simulink model "ofdm_txrx_alamouti"  (Simulink 6.3)
settings: Solver=ode45  StartTime=0.0  StopTime=45000  RelTol=1e-3
InitFcn:
  | ofdm_rx_alamouti_init
StartFcn:
  | ofdm_rx_alamouti_init
values from neighbouring files (symbols this model uses):
  CPLength = 16    [ofdm_tx_alamouti_init.m]
  CRCPolynomial = hex2dec('04c11db7')    [calcTxCRC.m]    (also in ofdm_tx_alamouti_init.m)
  hi = zeros(1,length(cci))    [ofdm_rx_alamouti_init.m]
  hr = zeros(1,length(ccr))    [ofdm_rx_alamouti_init.m]
  max_CP_length = 16    [ofdm_tx_alamouti_init.m]
  max_num_baseRateSymbols = 31    [ofdm_tx_alamouti_init.m]
  max_num_subcarriers = 64    [ofdm_tx_alamouti_init.m]
  max_num_trainingSymbols = 15    [ofdm_tx_alamouti_init.m]
  max_OFDM_symbols = 2047    [ofdm_tx_alamouti_init.m]
  modConstellation_qam16 = 0.75*[1 3 -1 -3]./3    [ofdm_tx_alamouti_init.m]
  modConstellation_qam256 = 0.9375*[3 1 5 7 11 9 13 15 -3 -1 -5 -7 -11 -9 -13 -15]./15    [ofdm_tx_alamouti_init.m]
  modConstellation_qam64 = 0.875*[3 1 5 7 -3 -1 -5 -7]./7    [ofdm_tx_alamouti_init.m]
  modConstellation_qpsk = [1 -1]./sqrt(2)    [ofdm_tx_alamouti_init.m]
  numBaseRateSymbols = 2    [ofdm_tx_alamouti_init.m]
  numFullRateSymbols = numFullRateSymbols + mod(numFullRateSymbols, 2)    [ofdm_tx_alamouti_init.m]
  numSubcarriers = 64    [ofdm_tx_alamouti_init.m]
  numTrainingSymbols = 4    [ofdm_tx_alamouti_init.m]
  pkt_crossCorr_thresh = 0.7    [ofdm_rx_alamouti_init.m]
  pkt_energy_thresh = 0    [ofdm_rx_alamouti_init.m]
  pkt_numPayloadBytes = 24+4+20+20+500    (= 568)    [ofdm_tx_alamouti_init.m]
  pktTiming_count_payloadStart = pktTiming_count_lsStart + pktTiming_ls_num*pktTiming_ls_length    [ofdm_rx_init_packetTimingControl.m]
  preamble = 6*[0 shortsyms_10 longsyms_2]    [ofdm_tx_alamouti_init.m]
  preamble_I = real(preamble)    [ofdm_tx_alamouti_init.m]
  preamble_Q = imag(preamble)    [ofdm_tx_alamouti_init.m]
  RAM_init_size = 4096    [ofdm_tx_alamouti_init.m]
  rx_longCorrCountLoad = 251-16    (= 235)    [ofdm_rx_alamouti_init.m]
  rx_longCorrThresh = 3000    [ofdm_rx_alamouti_init.m]
  RxDataScrambling_Seq = [40 198 78 63 82 173 102 245 48 111 172 115 147 230 216 93 72 65 62 2 205 242 122 90 128 83 105 97 73 10 5 252]    [ofdm_tx_alamouti_init.m]
  simOnly_numSamples = length(preamble)+( (numSubcarriers+CPLength)*(numTrainingSymbols + numBaseRateSymbols + numFullRateSymbols) )    [ofdm_tx_alamouti_init.m]
  train = [train train]    [ofdm_tx_alamouti_init.m]
  TxDataScrambling_Seq = [40 198 78 63 82 173 102 245 48 111 172 115 147 230 216 93 72 65 62 2 205 242 122 90 128 83 105 97 73 10 5 252]    [ofdm_tx_alamouti_init.m]
undefined symbols (not defined in the file or in its folder): CRC_table_gen, gcbh, interpFilter_4, xlBlockLoadCallback
libraries: xbsIndex_r4 x4373, dspmlti4 x4, dspsigops x4, commchan3 x2, simulink x2, commrflib2 x2, commsink2 x2, dspsrcs4 x1
(part 4 of 39)

== system ofdm_txrx_alamouti/OFDM Tx Alamouti/PreCFO ==
  " AI" : Inport
  " AQ" : Inport  Port=2
  " BI" : Inport  Port=3
  " BQ" : Inport  Port=4
  A*B : Subsystem
  A*B1 : Subsystem
  Convert{1..4} (4 alike) : xbsIndex_r4/Convert  arith_type="Signed  (2's comp)"  n_bits=16  bin_pt=15  quantization=Truncate  overflow=Flag as error  latency=0  dbl_ovrd=off  pipeline=off  block_type=convert
      settings: xl_area=[0 0 0 0 0 0 0]  block_version=VER_STRING_GOES_HERE
  Convert5 : xbsIndex_r4/Convert  arith_type=Unsigned  n_bits=32  bin_pt=32  quantization=Truncate  overflow=Wrap  latency=0  dbl_ovrd=off  pipeline=off  block_type=convert
      settings: xl_area=[0 0 0 0 0 0 0]  block_version=VER_STRING_GOES_HERE
  Convert6 : xbsIndex_r4/Convert  arith_type=Boolean  n_bits=32  bin_pt=32  quantization=Truncate  overflow=Wrap  latency=0  dbl_ovrd=off  pipeline=off  block_type=convert
      settings: xl_area=[0 0 0 0 0 0 0]  block_version=VER_STRING_GOES_HERE
  DDS : Subsystem
  Down Sample1 : xbsIndex_r4/Down Sample  sample_ratio=2  sample_phase="Last Value of Frame  (most efficient)"  en=off  latency=1  dbl_ovrd=off  block_type=dsamp
      settings: block_version=VER_STRING_GOES_HERE
  Freq Correction Sinusoids : Scope  NumInputPorts=2
      settings: TimeRange=5000  YMin=-1~-1  YMax=0.4~1  SaveName=ScopeData60
  Gateway Out : xbsIndex_r4/Gateway Out  hdl_port=off  timing_constraint=None  locs_specified=off  LOCs={}  block_type=gatewayout
      settings: infoedit="Gateway out block.  Converts Xilinx fixed point inputs into ouputs of type Simulink integer, double, or fixed point.<P><P>Hardware notes:  In hardware these blocks become top level output ports or are discarded, depending on how they are configured."  xl_area=[0 0 0 0 0 0 0]  block_version=VER_STRING_GOES_HERE
  Gateway Out1 : xbsIndex_r4/Gateway Out  hdl_port=off  timing_constraint=None  locs_specified=off  LOCs={}  block_type=gatewayout
      settings: infoedit="Gateway out block.  Converts Xilinx fixed point inputs into ouputs of type Simulink integer, double, or fixed point.<P><P>Hardware notes:  In hardware these blocks become top level output ports or are discarded, depending on how they are configured."  xl_area=[0 0 0 0 0 0 0]  block_version=VER_STRING_GOES_HERE
  Gateway Out2 : xbsIndex_r4/Gateway Out  hdl_port=off  timing_constraint=None  locs_specified=off  LOCs={}  block_type=gatewayout
      settings: infoedit="Gateway out block.  Converts Xilinx fixed point inputs into ouputs of type Simulink integer, double, or fixed point.<P><P>Hardware notes:  In hardware these blocks become top level output ports or are discarded, depending on how they are configured."  xl_area=[0 0 0 0 0 0 0]  block_version=VER_STRING_GOES_HERE
  Inverter : xbsIndex_r4/Inverter  en=off  latency=0  dbl_ovrd=off  block_type=inv
      settings: block_version=8.2.01
  Logical5 : xbsIndex_r4/Logical  logical_function=OR  inputs=2  en=off  latency=0  precision=Full  arith_type=Unsigned  n_bits=8  bin_pt=2  align_bp=on  dbl_ovrd=off  block_type=logical
      settings: xl_area=[0, 0, 0, 0, 0, 0, 0]  block_version=VER_STRING_GOES_HERE
  Mux2 : Mux  Inputs=2
  AI : Outport
  AQ : Outport  Port=2
  BI : Outport  Port=3
  BQ : Outport  Port=4
  wires:
  A*B1/2(Im) -> Convert4/1
  " BQ"/1 -> A*B1/2(Im[A])
  A*B1/1(Re) -> Convert3/1
  " AQ"/1 -> A*B/2(Im[A])
  A*B/1(Re) -> Convert1/1
  " AI"/1 -> A*B/1(Re[A])
  A*B/2(Im) -> Convert2/1
  Convert1/1 -> AI/1
  Convert2/1 -> AQ/1
  " BI"/1 -> A*B1/1(Re[A])
  Convert3/1 -> BI/1
  Convert4/1 -> BQ/1
  DDS/1(Q) -> A*B/3(Im[B]), A*B1/3(Im[B]), Gateway Out/1
  DDS/2(I) -> Gateway Out1/1, A*B/4(Re[B]), A*B1/4(Re[B])
  [Rx_CFO_PhaseInc] -> Convert5/1
  Convert5/1 -> DDS/1(PhaseInc), Gateway Out2/1
  [TxReset] -> Logical5/1
  Gateway Out1/1 -> Mux2/1
  Gateway Out/1 -> Mux2/2
  Mux2/1 -> Freq Correction Sinusoids/1
  Logical5/1 -> Down Sample1/1
  [TxReg_EnableTxPreCFO] -> Inverter/1
  Convert6/1 -> Logical5/2
  Down Sample1/1 -> DDS/2(Rst)
  Inverter/1 -> Convert6/1
  Gateway Out2/1 -> Freq Correction Sinusoids/2

== system ofdm_txrx_alamouti/OFDM Tx Alamouti/Restarter ==
  StartTx : Inport
  Done : Inport  Port=2
  Convert{,1..5} (6 alike) : xbsIndex_r4/Convert  arith_type=Boolean  n_bits=1  bin_pt=0  quantization=Truncate  overflow=Wrap  latency=0  dbl_ovrd=off  pipeline=off  block_type=convert
      settings: block_version=8.2.02
  Counter : xbsIndex_r4/Counter  cnt_type=Free Running  cnt_to=Inf  operation=Up  start_count=0  cnt_by_val=1  arith_type=Unsigned  n_bits=14  bin_pt=0  load_pin=off  rst=on  en=on  explicit_period=off  period=4  dbl_ovrd=off  use_behavioral_HDL=off  use_rpm=off  block_type=counter
      settings: block_version=8.2.02
  Delay : xbsIndex_r4/Delay  en=off  latency=16  dbl_ovrd=off  reg_retiming=off  block_type=delay
      settings: infoedit=Hardware notes: A delay line is a chain, each link of which is an SRL16 followed by a flip-flop. If register retiming is enabled, the delay line is a chain of flip-flops.  xl_area=[1 1 0 0 0 0 0]  block_version=VER_STRING_GOES_HERE
  Inverter : xbsIndex_r4/Inverter  en=off  latency=0  dbl_ovrd=off  block_type=inv
      settings: block_version=8.2.02
  Inverter1 : xbsIndex_r4/Inverter  en=off  latency=0  dbl_ovrd=off  block_type=inv
      settings: block_version=8.2.02
  Logical1 : xbsIndex_r4/Logical  logical_function=OR  inputs=3  en=off  latency=0  precision=Full  arith_type=Unsigned  n_bits=16  bin_pt=0  align_bp=on  dbl_ovrd=off  block_type=logical
      settings: block_version=VER_STRING_GOES_HERE
  Logical10 : xbsIndex_r4/Logical  logical_function=AND  inputs=2  en=off  latency=0  precision=Full  arith_type=Unsigned  n_bits=16  bin_pt=0  align_bp=on  dbl_ovrd=off  block_type=logical
      settings: block_version=VER_STRING_GOES_HERE
  Logical11 : xbsIndex_r4/Logical  logical_function=AND  inputs=2  en=off  latency=0  precision=Full  arith_type=Unsigned  n_bits=16  bin_pt=0  align_bp=on  dbl_ovrd=off  block_type=logical
      settings: block_version=VER_STRING_GOES_HERE
  Logical2 : xbsIndex_r4/Logical  logical_function=AND  inputs=3  en=off  latency=0  precision=Full  arith_type=Unsigned  n_bits=16  bin_pt=0  align_bp=on  dbl_ovrd=off  block_type=logical
      settings: block_version=VER_STRING_GOES_HERE
  Logical3 : xbsIndex_r4/Logical  logical_function=AND  inputs=2  en=off  latency=0  precision=Full  arith_type=Unsigned  n_bits=16  bin_pt=0  align_bp=on  dbl_ovrd=off  block_type=logical
      settings: block_version=VER_STRING_GOES_HERE
  Logical4 : xbsIndex_r4/Logical  logical_function=OR  inputs=3  en=off  latency=0  precision=Full  arith_type=Unsigned  n_bits=16  bin_pt=0  align_bp=on  dbl_ovrd=off  block_type=logical
      settings: block_version=VER_STRING_GOES_HERE
  Logical5 : xbsIndex_r4/Logical  logical_function=OR  inputs=2  en=off  latency=0  precision=Full  arith_type=Unsigned  n_bits=16  bin_pt=0  align_bp=on  dbl_ovrd=off  block_type=logical
      settings: block_version=VER_STRING_GOES_HERE
  Logical6 : xbsIndex_r4/Logical  logical_function=XOR  inputs=2  en=off  latency=0  precision=Full  arith_type=Unsigned  n_bits=16  bin_pt=0  align_bp=on  dbl_ovrd=off  block_type=logical
      settings: block_version=VER_STRING_GOES_HERE
  Logical7 : xbsIndex_r4/Logical  logical_function=OR  inputs=2  en=off  latency=0  precision=Full  arith_type=Unsigned  n_bits=16  bin_pt=0  align_bp=on  dbl_ovrd=off  block_type=logical
      settings: block_version=VER_STRING_GOES_HERE
  Logical8 : xbsIndex_r4/Logical  logical_function=AND  inputs=3  en=off  latency=0  precision=Full  arith_type=Unsigned  n_bits=16  bin_pt=0  align_bp=on  dbl_ovrd=off  block_type=logical
      settings: block_version=VER_STRING_GOES_HERE
  Posedge1 : Subsystem
  Posedge2 : Subsystem
  Radio_RxEn : xbsIndex_r4/Gateway Out  hdl_port=on  timing_constraint=None  locs_specified=off  LOCs={}  block_type=gatewayout
      settings: infoedit="Gateway out block.  Converts Xilinx fixed point inputs into ouputs of type Simulink integer, double, or fixed point.<P><P>Hardware notes:  In hardware these blocks become top level output ports or are discarded, depending on how they are configured."  xl_area=[0 0 0 0 1 0 0]  block_version=VER_STRING_GOES_HERE
  Radio_TxEn : xbsIndex_r4/Gateway Out  hdl_port=on  timing_constraint=None  locs_specified=off  LOCs={}  block_type=gatewayout
      settings: infoedit="Gateway out block.  Converts Xilinx fixed point inputs into ouputs of type Simulink integer, double, or fixed point.<P><P>Hardware notes:  In hardware these blocks become top level output ports or are discarded, depending on how they are configured."  xl_area=[0 0 0 0 1 0 0]  block_version=VER_STRING_GOES_HERE
  ReTx Control : Scope  NumInputPorts=8
      settings: TimeRange=7000  YMin=0~0~0~0~-1~-1~-1~-1  YMax=40~1~1~1~1~1~1~1  SaveName=ScopeData70
  Relational : xbsIndex_r4/Relational  mode=a=b  en=off  latency=1  dbl_ovrd=off  block_type=relational
      settings: block_version=8.2.02
  SR : Subsystem
  SR1 : Subsystem
  SR2 : Subsystem
  SR3 : Subsystem
  done{1..6} (6 alike) : xbsIndex_r4/Gateway Out  hdl_port=off  timing_constraint=None  locs_specified=off  LOCs={}  block_type=gatewayout
      settings: infoedit="Gateway out block.  Converts Xilinx fixed point inputs into ouputs of type Simulink integer, double, or fixed point.<P><P>Hardware notes:  In hardware these blocks become top level output ports or are discarded, depending on how they are configured."  xl_area=[0 0 0 0 1 0 0]  block_version=VER_STRING_GOES_HERE
  negedge : Subsystem
  Start : Outport
  2ndTx : Outport  Port=2
  tag scope: TxRestarter_DelayPktDone=global
  wires:
  Counter/1 -> Relational/2
  [TxReg_2ndTxDelay] -> Relational/1
  Logical1/1 -> SR/1(R)
  StartTx/1 -> done4/1, Posedge2/1(D)
  [TxReset] -> Logical1/3
  SR/1(Q) -> Counter/2, Inverter/1
  Posedge1/1(Q) -> Logical2/1, Logical5/2, SR2/2(R)
  [TxReg_2ndTxEn] -> Convert1/1
  Inverter/1 -> Counter/1
  Relational/1 -> Logical1/1, Logical3/1, Logical11/1
  [TxReg_2ndTxEn] -> Convert/1
  Logical3/1 -> Logical4/2, SR1/1(S)
  Logical4/1 -> Start/1, done1/1
  Convert1/1 -> Logical2/2, Logical10/1
  Logical5/1 -> SR1/2(R)
  [TxReset] -> Logical5/1
  SR1/1(Q) -> Logical6/1, Inverter1/1
  Logical6/1 -> 2ndTx/1, done2/1
  [TxReg_2ndTxAntB] -> Convert2/1
  Convert2/1 -> Logical6/2
  Inverter1/1 -> Logical2/3, Logical8/1, Logical10/2
  Done/1 -> Posedge1/1(D), done6/1
  done4/1 -> ReTx Control/1  "Ext Start"
  done1/1 -> ReTx Control/2  "Start Out"
  done2/1 -> ReTx Control/3  "2nd Tx Out"
  done3/1 -> ReTx Control/4  "DF Relay ReTx Start"
  done5/1 -> ReTx Control/5  "AF Relay ReTx Start"
  done6/1 -> ReTx Control/6  "Tx Done"
  Radio_RxEn/1 -> ReTx Control/8  "Radio_RxEn"
  [Rx_GoodPktInterrupt] -> Convert4/1
  [TxReg_RelayTxEn] -> Convert3/1
  Logical2/1 -> Logical7/1, done5/1
  Logical8/1 -> Logical7/2, done3/1
  Logical7/1 -> SR/2(S)
  Convert/1 -> Logical3/2
  Convert3/1 -> Logical8/2
  Convert4/1 -> Logical8/3
  [TxReg_RelayTxEn] -> Convert5/1
  Convert5/1 -> Logical11/2
  Posedge2/1(Q) -> Logical4/1, Logical1/2
  Logical10/1 -> [TxRestarter_DelayPktDone]
  SR2/1(Q) -> Radio_TxEn/1, negedge/1(D)
  Logical11/1 -> Logical4/3, SR2/1(S), SR3/2(R)
  negedge/1(Q) -> Delay/1
  Delay/1 -> SR3/1(S)
  SR3/1(Q) -> Radio_RxEn/1
  Radio_TxEn/1 -> ReTx Control/7  "Radio_TxEn"

== system ofdm_txrx_alamouti/OFDM Tx Alamouti/Training_Data ==
  CPn_Data : Inport
  train_pay : Inport  Port=2
  BaseRate_En : Inport  Port=3
  FFT En : Inport  Port=4
  start : Inport  Port=5
  packet_done : Inport  Port=6
  xn_index : Inport  Port=7
  Ant Selector : Subsystem
  Convert2 : xbsIndex_r4/Convert  arith_type="Signed  (2's comp)"  n_bits=16  bin_pt=15  quantization=Truncate  overflow=Wrap  latency=0  dbl_ovrd=off  pipeline=off  block_type=convert
      settings: xl_area=[0 0 0 0 0 0 0]  block_version=VER_STRING_GOES_HERE
  Convert3 : xbsIndex_r4/Convert  arith_type="Signed  (2's comp)"  n_bits=16  bin_pt=15  quantization=Truncate  overflow=Wrap  latency=0  dbl_ovrd=off  pipeline=off  block_type=convert
      settings: xl_area=[0 0 0 0 0 0 0]  block_version=VER_STRING_GOES_HERE
  Delay2 : xbsIndex_r4/Delay  en=off  latency=1  dbl_ovrd=off  reg_retiming=off  block_type=delay
      settings: infoedit=Hardware notes: A delay line is a chain, each link of which is an SRL16 followed by a flip-flop. If register retiming is enabled, the delay line is a chain of flip-flops.  xl_area=[1 1 0 0 0 0 0]  block_version=VER_STRING_GOES_HERE
  FlexibleMod : Subsystem
  Logical : xbsIndex_r4/Logical  logical_function=AND  inputs=3  en=off  latency=0  precision=Full  arith_type=Unsigned  n_bits=8  bin_pt=2  align_bp=on  dbl_ovrd=off  block_type=logical
      settings: xl_area=[0, 0, 0, 0, 0, 0, 0]  block_version=VER_STRING_GOES_HERE
  Logical1 : xbsIndex_r4/Logical  logical_function=OR  inputs=2  en=off  latency=0  precision=Full  arith_type=Unsigned  n_bits=8  bin_pt=2  align_bp=on  dbl_ovrd=off  block_type=logical
      settings: xl_area=[0, 0, 0, 0, 0, 0, 0]  block_version=VER_STRING_GOES_HERE
  Logical5 : xbsIndex_r4/Logical  logical_function=AND  inputs=2  en=off  latency=0  precision=Full  arith_type=Unsigned  n_bits=8  bin_pt=2  align_bp=on  dbl_ovrd=off  block_type=logical
      settings: xl_area=[0, 0, 0, 0, 0, 0, 0]  block_version=VER_STRING_GOES_HERE
  Training_Pilots : Subsystem
  tx_Q : Outport
  tx_I : Outport  Port=2
  wires:
  FlexibleMod/2(Q) -> Training_Pilots/3(Q_mod)
  FlexibleMod/1(I) -> Training_Pilots/2(I_mod)
  packet_done/1 -> FlexibleMod/2(packet_done), Logical1/1
  xn_index/1 -> Training_Pilots/1(Xn_index), FlexibleMod/1(ifft_index)
  train_pay/1 -> Delay2/1
  Logical/1 -> FlexibleMod/3(vin)
  start/1 -> FlexibleMod/4(start)
  BaseRate_En/1 -> Logical5/2
  CPn_Data/1 -> Ant Selector/1(CP#_Data), Logical/2
  Delay2/1 -> Logical5/1, Training_Pilots/4(train#), Logical/3
  Logical5/1 -> FlexibleMod/5(BaseRate_En)
  Ant Selector/1(Sel) -> Training_Pilots/5(antSel)
  FFT En/1 -> Logical/1
  Training_Pilots/1(tx_I) -> Convert3/1
  Training_Pilots/2(tx_Q) -> Convert2/1
  Convert3/1 -> tx_I/1
  Convert2/1 -> tx_Q/1
  Logical1/1 -> Training_Pilots/6(rst), Ant Selector/2(rst)
  [TxReset] -> Logical1/2

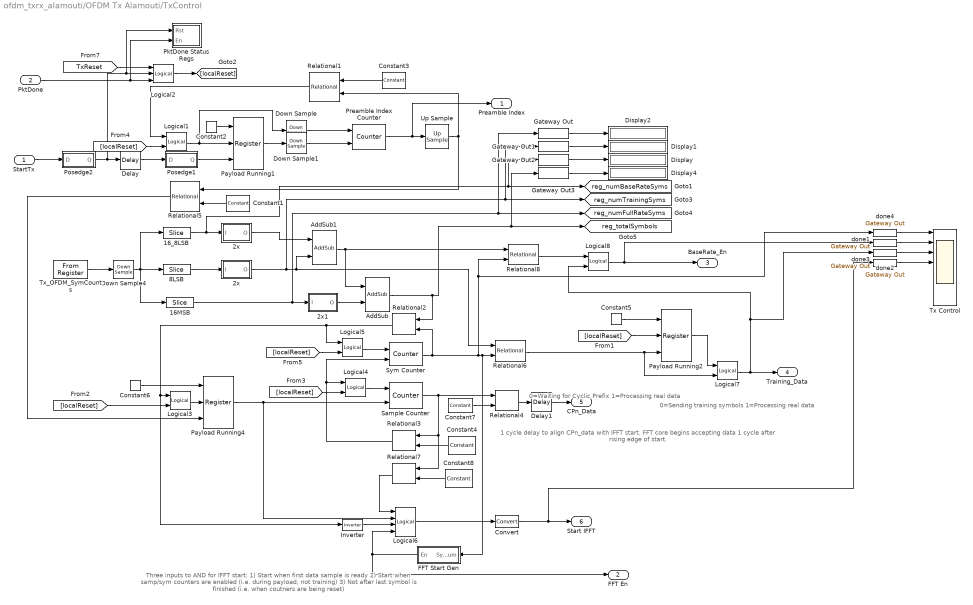
== system ofdm_txrx_alamouti/OFDM Tx Alamouti/TxControl ==
  note: 0=Waiting for Cyclic Prefix
      | 1=Processing real data
  note: 0=Sending training symbols
      | 1=Processing real data
  note: 1 cycle delay to align CPn_data
      | with IFFT start. FFT core begins
      | accepting data 1 cycle after
      | rising edge of start.
  note: Three inputs to AND for IFFT start:
      | 1) Start when first data sample is ready
      | 2) Start when samp/sym counters are enabled
      | (i.e. during payload, not training)
      | 3) Not after last symbol is finished
      | (i.e. when coutners are being reset)
  StartTx : Inport
  PktDone : Inport  Port=2
  16MSB : xbsIndex_r4/Slice  nbits=ceil(log2(max_OFDM_symbols))  boolean_output=off  mode=Lower Bit Location + Width  bit1=0  base1=MSB of Input  bit0=16  base0=LSB of Input  dbl_ovrd=off  block_type=slice
      settings: infoedit="Extracts a given range of bits from each input sample and presents it at the output.  The output type is ordinarily unsigned with binary point at zero, but can be Boolean when the slice is one bit wide.<P><P>Hardware notes: In hardware this block costs nothing."  block_version=VER_STRING_GOES_HERE
  16_8LSB : xbsIndex_r4/Slice  nbits=ceil(log2(max_num_baseRateSymbols+1))  boolean_output=off  mode=Lower Bit Location + Width  bit1=0  base1=MSB of Input  bit0=8  base0=LSB of Input  dbl_ovrd=off  block_type=slice
      settings: infoedit="Extracts a given range of bits from each input sample and presents it at the output.  The output type is ordinarily unsigned with binary point at zero, but can be Boolean when the slice is one bit wide.<P><P>Hardware notes: In hardware this block costs nothing."  block_version=VER_STRING_GOES_HERE
  2x : Subsystem
  "2x " : Subsystem
  2x1 : Subsystem
  8LSB : xbsIndex_r4/Slice  nbits=ceil(log2(max_num_trainingSymbols+1))  boolean_output=off  mode=Lower Bit Location + Width  bit1=0  base1=MSB of Input  bit0=0  base0=LSB of Input  dbl_ovrd=off  block_type=slice
      settings: infoedit="Extracts a given range of bits from each input sample and presents it at the output.  The output type is ordinarily unsigned with binary point at zero, but can be Boolean when the slice is one bit wide.<P><P>Hardware notes: In hardware this block costs nothing."  block_version=VER_STRING_GOES_HERE
  AddSub : xbsIndex_r4/AddSub  mode=Addition  use_carryin=off  use_carryout=off  en=off  latency=0  precision=Full  arith_type=Unsigned  n_bits=10  bin_pt=0  quantization=Truncate  overflow=Wrap  dbl_ovrd=off  use_behavioral_HDL=on  pipelined=off  use_rpm=on  block_type=addsub
      settings: xl_area=[0, 0, 0, 0, 0, 0, 0]  block_version=VER_STRING_GOES_HERE
  AddSub1 : xbsIndex_r4/AddSub  mode=Addition  use_carryin=off  use_carryout=off  en=off  latency=0  precision=Full  arith_type=Unsigned  n_bits=10  bin_pt=0  quantization=Truncate  overflow=Wrap  dbl_ovrd=off  use_behavioral_HDL=on  pipelined=off  use_rpm=on  block_type=addsub
      settings: xl_area=[0, 0, 0, 0, 0, 0, 0]  block_version=VER_STRING_GOES_HERE
  Constant1 : xbsIndex_r4/Constant  Orientation=left  arith_type=Unsigned  const=length(preamble)-158  n_bits=ceil(log2(length(preamble)))  bin_pt=0  explicit_period=off  period=1  equ=P=C  opselect=C  inp2=PCIN>>17  opr=+  inp1=P  carry=CIN  dbl_ovrd=off  block_type=constant
      settings: block_version=VER_STRING_GOES_HERE
  Constant2 : xbsIndex_r4/Constant  arith_type=Boolean  const=1  n_bits=1  bin_pt=0  explicit_period=off  period=1  equ=P=C  opselect=C  inp2=PCIN>>17  opr=+  inp1=P  carry=CIN  dbl_ovrd=off  block_type=constant
      settings: block_version=VER_STRING_GOES_HERE
  Constant3 : xbsIndex_r4/Constant  Orientation=left  arith_type=Unsigned  const=length(preamble)-1  n_bits=ceil(log2(length(preamble)))  bin_pt=0  explicit_period=off  period=1  equ=P=C  opselect=C  inp2=PCIN>>17  opr=+  inp1=P  carry=CIN  dbl_ovrd=off  block_type=constant
      settings: block_version=VER_STRING_GOES_HERE
  Constant4 : xbsIndex_r4/Constant  Orientation=left  arith_type=Unsigned  const=numSubcarriers+CPLength-1  n_bits=ceil(log2(max_num_subcarriers+CPLength+1))  bin_pt=0  explicit_period=off  period=1  equ=P=C  opselect=C  inp2=PCIN>>17  opr=+  inp1=P  carry=CIN  dbl_ovrd=off  block_type=constant
      settings: block_version=VER_STRING_GOES_HERE
  Constant5 : xbsIndex_r4/Constant  arith_type=Boolean  const=1  n_bits=1  bin_pt=0  explicit_period=off  period=1  equ=P=C  opselect=C  inp2=PCIN>>17  opr=+  inp1=P  carry=CIN  dbl_ovrd=off  block_type=constant
      settings: block_version=VER_STRING_GOES_HERE
  Constant6 : xbsIndex_r4/Constant  arith_type=Boolean  const=1  n_bits=1  bin_pt=0  explicit_period=off  period=1  equ=P=C  opselect=C  inp2=PCIN>>17  opr=+  inp1=P  carry=CIN  dbl_ovrd=off  block_type=constant
      settings: block_version=VER_STRING_GOES_HERE
  Constant7 : xbsIndex_r4/Constant  arith_type=Unsigned  const=numSubcarriers-1  n_bits=log2(numSubcarriers)  bin_pt=0  explicit_period=off  period=1  equ=P=C  opselect=C  inp2=PCIN>>17  opr=+  inp1=P  carry=CIN  dbl_ovrd=off  block_type=constant
      settings: block_version=VER_STRING_GOES_HERE
  Constant8 : xbsIndex_r4/Constant  Orientation=left  arith_type=Unsigned  const=0  n_bits=1  bin_pt=0  explicit_period=off  period=1  equ=P=C  opselect=C  inp2=PCIN>>17  opr=+  inp1=P  carry=CIN  dbl_ovrd=off  block_type=constant
      settings: block_version=VER_STRING_GOES_HERE
  Convert : xbsIndex_r4/Convert  arith_type=Boolean  n_bits=8  bin_pt=6  quantization=Truncate  overflow=Wrap  latency=0  dbl_ovrd=off  pipeline=off  block_type=convert
      settings: xl_area=[0, 0, 0, 0, 0, 0, 0]  block_version=VER_STRING_GOES_HERE
  Delay : xbsIndex_r4/Delay  en=off  latency=1  dbl_ovrd=off  reg_retiming=off  block_type=delay
      settings: infoedit=Hardware notes: A delay line is a chain, each link of which is an SRL16 followed by a flip-flop. If register retiming is enabled, the delay line is a chain of flip-flops.  xl_area=[1 1 0 0 0 0 0]  block_version=VER_STRING_GOES_HERE
  Delay1 : xbsIndex_r4/Delay  en=off  latency=1  dbl_ovrd=off  reg_retiming=off  block_type=delay
      settings: infoedit=Hardware notes: A delay line is a chain, each link of which is an SRL16 followed by a flip-flop. If register retiming is enabled, the delay line is a chain of flip-flops.  xl_area=[1 1 0 0 0 0 0]  block_version=VER_STRING_GOES_HERE
  Display{,1..2,4} (4 alike) : Display
  Down Sample : xbsIndex_r4/Down Sample  sample_ratio=2  sample_phase=First Value of Frame  en=off  latency=0  dbl_ovrd=off  block_type=dsamp
      settings: block_version=VER_STRING_GOES_HERE
  Down Sample1 : xbsIndex_r4/Down Sample  sample_ratio=2  sample_phase=First Value of Frame  en=off  latency=0  dbl_ovrd=off  block_type=dsamp
      settings: block_version=VER_STRING_GOES_HERE
  Down Sample4 : xbsIndex_r4/Down Sample  sample_ratio=2  sample_phase="Last Value of Frame  (most efficient)"  en=off  latency=1  dbl_ovrd=off  block_type=dsamp
      settings: block_version=VER_STRING_GOES_HERE
  FFT Start Gen : Subsystem  Orientation=left
  Gateway Out{,1..3} (4 alike) : xbsIndex_r4/Gateway Out  hdl_port=off  timing_constraint=None  locs_specified=off  LOCs={}  block_type=gatewayout
      settings: infoedit="Gateway out block.  Converts Xilinx fixed point inputs into ouputs of type Simulink integer, double, or fixed point.<P><P>Hardware notes:  In hardware these blocks become top level output ports or are discarded, depending on how they are configured."  block_version=VER_STRING_GOES_HERE
  Inverter : xbsIndex_r4/Inverter  en=off  latency=0  dbl_ovrd=off  block_type=inv
      settings: xl_area=[0, 0, 0, 0, 0, 0, 0]  block_version=VER_STRING_GOES_HERE
  Logical{1,3..5,7} (5 alike) : xbsIndex_r4/Logical  logical_function=OR  inputs=2  en=off  latency=0  precision=Full  arith_type=Unsigned  n_bits=8  bin_pt=2  align_bp=on  dbl_ovrd=off  block_type=logical
      settings: xl_area=[1 0 0 1 0 0 0]  block_version=VER_STRING_GOES_HERE
  Logical2 : xbsIndex_r4/Logical  logical_function=OR  inputs=3  en=off  latency=0  precision=Full  arith_type=Unsigned  n_bits=8  bin_pt=2  align_bp=on  dbl_ovrd=off  block_type=logical
      settings: xl_area=[1 0 0 1 0 0 0]  block_version=VER_STRING_GOES_HERE
  Logical6 : xbsIndex_r4/Logical  logical_function=AND  inputs=4  en=off  latency=0  precision=Full  arith_type=Unsigned  n_bits=1  bin_pt=0  align_bp=on  dbl_ovrd=off  block_type=logical
      settings: xl_area=[1 0 0 1 0 0 0]  block_version=VER_STRING_GOES_HERE
  Logical8 : xbsIndex_r4/Logical  logical_function=AND  inputs=2  en=off  latency=0  precision=Full  arith_type=Unsigned  n_bits=8  bin_pt=2  align_bp=on  dbl_ovrd=off  block_type=logical
      settings: xl_area=[1 0 0 1 0 0 0]  block_version=VER_STRING_GOES_HERE
  Payload Running1 : xbsIndex_r4/Register  init=0  rst=on  en=on  dbl_ovrd=off  block_type=register
      settings: xl_area=[1 1 0 0 0 0 0]  block_version=VER_STRING_GOES_HERE
  Payload Running2 : xbsIndex_r4/Register  init=0  rst=on  en=on  dbl_ovrd=off  block_type=register
      settings: xl_area=[1 1 0 0 0 0 0]  block_version=VER_STRING_GOES_HERE
  Payload Running4 : xbsIndex_r4/Register  init=0  rst=on  en=on  dbl_ovrd=off  block_type=register
      settings: xl_area=[1 1 0 0 0 0 0]  block_version=VER_STRING_GOES_HERE
  PktDone Status Regs : Subsystem
  Posedge1 : Subsystem
  Posedge2 : Subsystem
  Preamble Index Counter : xbsIndex_r4/Counter  cnt_type=Free Running  cnt_to=Inf  operation=Up  start_count=0  cnt_by_val=1  arith_type=Unsigned  n_bits=ceil(log2(1+length(preamble_Q)))  bin_pt=0  load_pin=off  rst=on  en=on  explicit_period=off  period=1  dbl_ovrd=off  use_behavioral_HDL=off  use_rpm=on  block_type=counter
      settings: xl_area=[5 9 0 9 0 0 0]  block_version=VER_STRING_GOES_HERE
  Relational{1,3,5,7} (4 alike) : xbsIndex_r4/Relational  Orientation=left  mode=a=b  en=off  latency=0  dbl_ovrd=off  block_type=relational
      Relational1 settings: xl_area=[3 0 0 5 0 0 0]  block_version=VER_STRING_GOES_HERE
      Relational3 settings: xl_area=[2 0 0 4 0 0 0]  block_version=VER_STRING_GOES_HERE
      Relational5 settings: xl_area=[3 0 0 5 0 0 0]  block_version=VER_STRING_GOES_HERE
      Relational7 settings: xl_area=[2 0 0 4 0 0 0]  block_version=VER_STRING_GOES_HERE
  Relational2 : xbsIndex_r4/Relational  Orientation=left  mode=a<=b  en=off  latency=0  dbl_ovrd=off  block_type=relational
      settings: xl_area=[2 0 0 4 0 0 0]  block_version=VER_STRING_GOES_HERE
  Relational4 : xbsIndex_r4/Relational  mode=a<=b  en=off  latency=0  dbl_ovrd=off  block_type=relational
      settings: xl_area=[2 0 0 4 0 0 0]  block_version=VER_STRING_GOES_HERE
  Relational6 : xbsIndex_r4/Relational  mode=a<=b  en=off  latency=0  dbl_ovrd=off  block_type=relational
      settings: xl_area=[2 0 0 4 0 0 0]  block_version=VER_STRING_GOES_HERE
  Relational8 : xbsIndex_r4/Relational  mode=a>b  en=off  latency=0  dbl_ovrd=off  block_type=relational
      settings: xl_area=[2 0 0 4 0 0 0]  block_version=VER_STRING_GOES_HERE
  Sample Counter : xbsIndex_r4/Counter  cnt_type=Free Running  cnt_to=Inf  operation=Up  start_count=0  cnt_by_val=1  arith_type=Unsigned  n_bits=1+ceil(log2(numSubcarriers + CPLength + 1))  bin_pt=0  load_pin=off  rst=on  en=on  explicit_period=off  period=1  dbl_ovrd=off  use_behavioral_HDL=off  use_rpm=on  block_type=counter
      settings: xl_area=[4 7 0 7 0 0 0]  block_version=VER_STRING_GOES_HERE
  Sym Counter : xbsIndex_r4/Counter  cnt_type=Free Running  cnt_to=Inf  operation=Up  start_count=0  cnt_by_val=1  arith_type=Unsigned  n_bits=ceil(log2(max_OFDM_symbols+1))  bin_pt=0  load_pin=off  rst=on  en=on  explicit_period=off  period=1  dbl_ovrd=off  use_behavioral_HDL=off  use_rpm=on  block_type=counter
      settings: xl_area=[4 7 0 7 0 0 0]  block_version=VER_STRING_GOES_HERE
  Tx Control : Scope  NumInputPorts=8
      settings: TimeRange=7000  YMin=0~0~0~0~-1~-1~-1~-1  YMax=40~1~1~1~1~1~1~1  SaveName=ScopeData61
  Tx_OFDM_SymCounts : xbsIndex_r4/From Register  shared_memory_name='Tx_OFDM_SymCounts'  init=(2^16 * numFullRateSymbols) + (2^8 *numBaseRateSymbols ) + numTrainingSymbols  period=1  ownership=Locally owned and initialized  arith_type=Unsigned  n_bits=32  bin_pt=0  dbl_ovrd=off  block_type=fromreg
      settings: AttributesFormatString=<< %<shared_memory_name> >>  infoedit="Register block that reads data to a shared memory register.  Delay of one sample period."  block_version=VER_STRING_GOES_HERE
  Up Sample : xbsIndex_r4/Up Sample  sample_ratio=2  copy_samples=on  dbl_ovrd=off  block_type=usamp
      settings: infoedit="Up samples input data.  Inserted values  can be zeros or copies of the most recent input sample.<P><P>Hardware notes: No hardware is needed if inserted values are copies of the input sample; otherwise, a mux and single bit flip-flop are used."  block_version=VER_STRING_GOES_HERE
  done{1..4} (4 alike) : xbsIndex_r4/Gateway Out  hdl_port=off  timing_constraint=None  locs_specified=off  LOCs={}  block_type=gatewayout
      settings: infoedit="Gateway out block.  Converts Xilinx fixed point inputs into ouputs of type Simulink integer, double, or fixed point.<P><P>Hardware notes:  In hardware these blocks become top level output ports or are discarded, depending on how they are configured."  xl_area=[0 0 0 0 1 0 0]  block_version=VER_STRING_GOES_HERE
  Preamble Index : Outport
  FFT En : Outport  Port=2
  BaseRate_En : Outport  Port=3
  Training_Data : Outport  Port=4
  CPn_Data : Outport  Port=5
  Start IFFT : Outport  Port=6
  tag scope: reg_numBaseRateSyms=global, reg_numFullRateSyms=global, reg_numTrainingSyms=global, reg_totalSymbols=global
  wires:
  Payload Running1/1 -> Down Sample1/1
  StartTx/1 -> Posedge2/1(D)
  Constant2/1 -> Payload Running1/1
  Logical1/1 -> Payload Running1/2, Down Sample/1
  [localReset] -> Logical1/2
  Preamble Index Counter/1 -> Up Sample/1, Preamble Index/1
  Relational1/1 -> Logical1/1
  Constant3/1 -> Relational1/1
  Posedge1/1(Q) -> Payload Running1/3
  Sample Counter/1 -> Relational4/1, Relational3/1, Relational7/1
  Relational3/1 -> Sym Counter/2, Logical4/1
  Constant6/1 -> Payload Running4/1
  Logical3/1 -> Payload Running4/2
  [localReset] -> Logical3/2
  Payload Running4/1 -> Sample Counter/2, Logical6/2
  Logical4/1 -> Sample Counter/1
  [localReset] -> Logical4/2
  Logical5/1 -> Sym Counter/1
  [localReset] -> Logical5/2
  Sym Counter/1 -> Relational2/2, Relational6/2, FFT Start Gen/1(SymNum), Relational8/2, done4/1
  Relational2/1 -> Logical3/1, Inverter/1, Logical5/1
  Relational4/1 -> Delay1/1
  Relational6/1 -> Payload Running2/3, Logical7/2
  Posedge2/1(Q) -> Delay/1, Logical2/2, PktDone Status Regs/1(Rst)
  Logical2/1 -> [localReset]
  Delay/1 -> Posedge1/1(D)
  Constant1/1 -> Relational5/2
  Relational5/1 -> Payload Running4/3
  [TxReset] -> Logical2/1
  Constant7/1 -> Relational4/2
  PktDone/1 -> Logical2/3, PktDone Status Regs/2(En)
  Constant5/1 -> Payload Running2/1
  [localReset] -> Payload Running2/2
  Payload Running2/1 -> Logical7/1
  Constant8/1 -> Relational7/2
  Relational7/1 -> Logical6/1
  Logical6/1 -> Convert/1
  Convert/1 -> Start IFFT/1, done3/1
  Logical7/1 -> Training_Data/1, done2/1, Logical8/2
  Delay1/1 -> CPn_Data/1
  Inverter/1 -> Logical6/3
  Relational8/1 -> Logical8/1
  AddSub1/1 -> Relational8/1, AddSub/1
  Constant4/1 -> Relational3/2
  FFT Start Gen/1(En) -> Logical6/4, FFT En/1
  "2x "/1(O) -> [reg_numTrainingSyms], Gateway Out1/1, Relational6/1, AddSub1/2
  2x/1(O) -> AddSub1/1
  AddSub/1 -> Relational2/1, [reg_totalSymbols], Gateway Out3/1
  Gateway Out/1 -> Display2/1
  Gateway Out1/1 -> Display1/1
  Gateway Out2/1 -> Display/1
  Gateway Out3/1 -> Display4/1
  Down Sample/1 -> Preamble Index Counter/1
  Down Sample1/1 -> Preamble Index Counter/2
  Up Sample/1 -> Relational5/1, Relational1/2
  16_8LSB/1 -> 2x/1(I), [reg_numBaseRateSyms], Gateway Out2/1
  8LSB/1 -> "2x "/1(I)
  Tx_OFDM_SymCounts/1 -> Down Sample4/1
  Down Sample4/1 -> 8LSB/1, 16_8LSB/1, 16MSB/1
  16MSB/1 -> [reg_numFullRateSyms], Gateway Out/1, 2x1/1(I)
  done4/1 -> Tx Control/1
  done1/1 -> Tx Control/2
  done2/1 -> Tx Control/3
  done3/1 -> Tx Control/4
  ? -> Tx Control/5
  ? -> Tx Control/6
  ? -> Tx Control/7
  ? -> Tx Control/8
  Logical8/1 -> done1/1, BaseRate_En/1
  2x1/1(O) -> AddSub/2

== system ofdm_txrx_alamouti/OFDM Tx Alamouti/TxStart ==
  note: Packet transmission can be triggered either by
      | software (writing a 1 to TxReg_TxStart) or
      | by asserting this top-level port. It is the application's
      | responsibility to gaurantee duplicate triggers aren't generated.
  Copy_Downsample : Subsystem
  Logical : xbsIndex_r4/Logical  logical_function=OR  inputs=2  en=off  latency=0  precision=Full  arith_type=Unsigned  n_bits=16  bin_pt=0  align_bp=on  dbl_ovrd=off  block_type=logical
      settings: block_version=VER_STRING_GOES_HERE
  Pulse Generator1 : DiscretePulseGenerator  Period=(simOnly_numSamples*2 + 500)*2*2  PhaseDelay=5
  Tx_StartTransmit : xbsIndex_r4/Gateway In  arith_type=Boolean  n_bits=1  bin_pt=0  quantization="Round  (unbiased: +/- Inf)"  overflow=Saturate  period=1  dbl_ovrd=off  timing_constraint=None  locs_specified=off  LOCs={}  block_type=gatewayin
      settings: infoedit="Gateway in block.  Converts inputs of type Simulink integer, double and fixed point to  Xilinx fixed point type.<P><P>Hardware notes:  In hardware these blocks become top level input ports."  xl_area=[0, 0, 0, 0, 0, 0, 0]  block_version=VER_STRING_GOES_HERE
  Start : Outport
  wires:
  Tx_StartTransmit/1 -> Copy_Downsample/1(D)
  Pulse Generator1/1 -> Tx_StartTransmit/1
  Logical/1 -> Start/1
  [TxReg_TxStart] -> Logical/2
  Copy_Downsample/1(Q) -> Logical/1

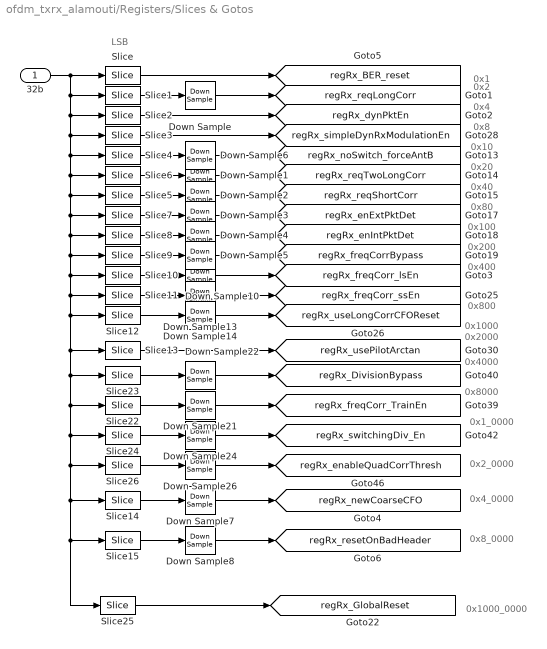
== system ofdm_txrx_alamouti/Registers/Slices & Gotos ==
  note: LSB
  note: 0x1
  note: 0x2
  note: 0x4
  note: 0x8
  note: 0x10
  note: 0x20
  note: 0x40
  note: 0x80
  note: 0x100
  note: 0x200
  note: 0x400
  note: 0x800
  note: 0x1000
  note: 0x2000
  note: 0x4000
  note: 0x8000
  note: 0x1_0000
  note: 0x1000_0000
  note: 0x2_0000
  note: 0x4_0000
  note: 0x8_0000
  32b : Inport
  Down Sample{,1..8,10,13..14,21..22,24,26} (16 alike) : xbsIndex_r4/Down Sample  sample_ratio=2  sample_phase="Last Value of Frame  (most efficient)"  en=off  latency=1  dbl_ovrd=off  block_type=dsamp
      settings: block_version=VER_STRING_GOES_HERE
  Slice : xbsIndex_r4/Slice  nbits=1  boolean_output=on  mode=Lower Bit Location + Width  bit1=0  base1=MSB of Input  bit0=0  base0=LSB of Input  dbl_ovrd=off  block_type=slice
      settings: infoedit="Extracts a given range of bits from each input sample and presents it at the output.  The output type is ordinarily unsigned with binary point at zero, but can be Boolean when the slice is one bit wide.<P><P>Hardware notes: In hardware this block costs nothing."  block_version=VER_STRING_GOES_HERE
  Slice1 : xbsIndex_r4/Slice  nbits=1  boolean_output=on  mode=Lower Bit Location + Width  bit1=0  base1=MSB of Input  bit0=1  base0=LSB of Input  dbl_ovrd=off  block_type=slice
      settings: infoedit="Extracts a given range of bits from each input sample and presents it at the output.  The output type is ordinarily unsigned with binary point at zero, but can be Boolean when the slice is one bit wide.<P><P>Hardware notes: In hardware this block costs nothing."  block_version=VER_STRING_GOES_HERE
  Slice10 : xbsIndex_r4/Slice  nbits=1  boolean_output=on  mode=Lower Bit Location + Width  bit1=0  base1=MSB of Input  bit0=10  base0=LSB of Input  dbl_ovrd=off  block_type=slice
      settings: infoedit="Extracts a given range of bits from each input sample and presents it at the output.  The output type is ordinarily unsigned with binary point at zero, but can be Boolean when the slice is one bit wide.<P><P>Hardware notes: In hardware this block costs nothing."  block_version=VER_STRING_GOES_HERE
  Slice11 : xbsIndex_r4/Slice  nbits=1  boolean_output=on  mode=Lower Bit Location + Width  bit1=0  base1=MSB of Input  bit0=11  base0=LSB of Input  dbl_ovrd=off  block_type=slice
      settings: infoedit="Extracts a given range of bits from each input sample and presents it at the output.  The output type is ordinarily unsigned with binary point at zero, but can be Boolean when the slice is one bit wide.<P><P>Hardware notes: In hardware this block costs nothing."  block_version=VER_STRING_GOES_HERE
  Slice12 : xbsIndex_r4/Slice  nbits=1  boolean_output=on  mode=Lower Bit Location + Width  bit1=0  base1=MSB of Input  bit0=12  base0=LSB of Input  dbl_ovrd=off  block_type=slice
      settings: infoedit="Extracts a given range of bits from each input sample and presents it at the output.  The output type is ordinarily unsigned with binary point at zero, but can be Boolean when the slice is one bit wide.<P><P>Hardware notes: In hardware this block costs nothing."  block_version=VER_STRING_GOES_HERE
  Slice13 : xbsIndex_r4/Slice  nbits=1  boolean_output=on  mode=Lower Bit Location + Width  bit1=0  base1=MSB of Input  bit0=13  base0=LSB of Input  dbl_ovrd=off  block_type=slice
      settings: infoedit="Extracts a given range of bits from each input sample and presents it at the output.  The output type is ordinarily unsigned with binary point at zero, but can be Boolean when the slice is one bit wide.<P><P>Hardware notes: In hardware this block costs nothing."  block_version=VER_STRING_GOES_HERE
  Slice14 : xbsIndex_r4/Slice  nbits=1  boolean_output=on  mode=Lower Bit Location + Width  bit1=0  base1=MSB of Input  bit0=18  base0=LSB of Input  dbl_ovrd=off  block_type=slice
      settings: infoedit="Extracts a given range of bits from each input sample and presents it at the output.  The output type is ordinarily unsigned with binary point at zero, but can be Boolean when the slice is one bit wide.<P><P>Hardware notes: In hardware this block costs nothing."  block_version=VER_STRING_GOES_HERE
  Slice15 : xbsIndex_r4/Slice  nbits=1  boolean_output=on  mode=Lower Bit Location + Width  bit1=0  base1=MSB of Input  bit0=19  base0=LSB of Input  dbl_ovrd=off  block_type=slice
      settings: infoedit="Extracts a given range of bits from each input sample and presents it at the output.  The output type is ordinarily unsigned with binary point at zero, but can be Boolean when the slice is one bit wide.<P><P>Hardware notes: In hardware this block costs nothing."  block_version=VER_STRING_GOES_HERE
  Slice2 : xbsIndex_r4/Slice  nbits=1  boolean_output=on  mode=Lower Bit Location + Width  bit1=0  base1=MSB of Input  bit0=2  base0=LSB of Input  dbl_ovrd=off  block_type=slice
      settings: infoedit="Extracts a given range of bits from each input sample and presents it at the output.  The output type is ordinarily unsigned with binary point at zero, but can be Boolean when the slice is one bit wide.<P><P>Hardware notes: In hardware this block costs nothing."  block_version=VER_STRING_GOES_HERE
  Slice22 : xbsIndex_r4/Slice  nbits=1  boolean_output=on  mode=Lower Bit Location + Width  bit1=0  base1=MSB of Input  bit0=15  base0=LSB of Input  dbl_ovrd=off  block_type=slice
      settings: infoedit="Extracts a given range of bits from each input sample and presents it at the output.  The output type is ordinarily unsigned with binary point at zero, but can be Boolean when the slice is one bit wide.<P><P>Hardware notes: In hardware this block costs nothing."  block_version=VER_STRING_GOES_HERE
  Slice23 : xbsIndex_r4/Slice  nbits=1  boolean_output=on  mode=Lower Bit Location + Width  bit1=0  base1=MSB of Input  bit0=14  base0=LSB of Input  dbl_ovrd=off  block_type=slice
      settings: infoedit="Extracts a given range of bits from each input sample and presents it at the output.  The output type is ordinarily unsigned with binary point at zero, but can be Boolean when the slice is one bit wide.<P><P>Hardware notes: In hardware this block costs nothing."  block_version=VER_STRING_GOES_HERE
  Slice24 : xbsIndex_r4/Slice  nbits=1  boolean_output=on  mode=Lower Bit Location + Width  bit1=0  base1=MSB of Input  bit0=16  base0=LSB of Input  dbl_ovrd=off  block_type=slice
      settings: infoedit="Extracts a given range of bits from each input sample and presents it at the output.  The output type is ordinarily unsigned with binary point at zero, but can be Boolean when the slice is one bit wide.<P><P>Hardware notes: In hardware this block costs nothing."  block_version=VER_STRING_GOES_HERE
  Slice25 : xbsIndex_r4/Slice  nbits=1  boolean_output=on  mode=Upper Bit Location + Width  bit1=0  base1=MSB of Input  bit0=31  base0=LSB of Input  dbl_ovrd=off  block_type=slice
      settings: infoedit="Extracts a given range of bits from each input sample and presents it at the output.  The output type is ordinarily unsigned with binary point at zero, but can be Boolean when the slice is one bit wide.<P><P>Hardware notes: In hardware this block costs nothing."  block_version=VER_STRING_GOES_HERE
  Slice26 : xbsIndex_r4/Slice  nbits=1  boolean_output=on  mode=Lower Bit Location + Width  bit1=0  base1=MSB of Input  bit0=17  base0=LSB of Input  dbl_ovrd=off  block_type=slice
      settings: infoedit="Extracts a given range of bits from each input sample and presents it at the output.  The output type is ordinarily unsigned with binary point at zero, but can be Boolean when the slice is one bit wide.<P><P>Hardware notes: In hardware this block costs nothing."  block_version=VER_STRING_GOES_HERE
  Slice3 : xbsIndex_r4/Slice  nbits=1  boolean_output=on  mode=Lower Bit Location + Width  bit1=0  base1=MSB of Input  bit0=3  base0=LSB of Input  dbl_ovrd=off  block_type=slice
      settings: infoedit="Extracts a given range of bits from each input sample and presents it at the output.  The output type is ordinarily unsigned with binary point at zero, but can be Boolean when the slice is one bit wide.<P><P>Hardware notes: In hardware this block costs nothing."  block_version=VER_STRING_GOES_HERE
  Slice4 : xbsIndex_r4/Slice  nbits=1  boolean_output=on  mode=Lower Bit Location + Width  bit1=0  base1=MSB of Input  bit0=4  base0=LSB of Input  dbl_ovrd=off  block_type=slice
      settings: infoedit="Extracts a given range of bits from each input sample and presents it at the output.  The output type is ordinarily unsigned with binary point at zero, but can be Boolean when the slice is one bit wide.<P><P>Hardware notes: In hardware this block costs nothing."  block_version=VER_STRING_GOES_HERE
  Slice5 : xbsIndex_r4/Slice  nbits=1  boolean_output=on  mode=Lower Bit Location + Width  bit1=0  base1=MSB of Input  bit0=6  base0=LSB of Input  dbl_ovrd=off  block_type=slice
      settings: infoedit="Extracts a given range of bits from each input sample and presents it at the output.  The output type is ordinarily unsigned with binary point at zero, but can be Boolean when the slice is one bit wide.<P><P>Hardware notes: In hardware this block costs nothing."  block_version=VER_STRING_GOES_HERE
  Slice6 : xbsIndex_r4/Slice  nbits=1  boolean_output=on  mode=Lower Bit Location + Width  bit1=0  base1=MSB of Input  bit0=5  base0=LSB of Input  dbl_ovrd=off  block_type=slice
      settings: infoedit="Extracts a given range of bits from each input sample and presents it at the output.  The output type is ordinarily unsigned with binary point at zero, but can be Boolean when the slice is one bit wide.<P><P>Hardware notes: In hardware this block costs nothing."  block_version=VER_STRING_GOES_HERE
  Slice7 : xbsIndex_r4/Slice  nbits=1  boolean_output=on  mode=Lower Bit Location + Width  bit1=0  base1=MSB of Input  bit0=7  base0=LSB of Input  dbl_ovrd=off  block_type=slice
      settings: infoedit="Extracts a given range of bits from each input sample and presents it at the output.  The output type is ordinarily unsigned with binary point at zero, but can be Boolean when the slice is one bit wide.<P><P>Hardware notes: In hardware this block costs nothing."  block_version=VER_STRING_GOES_HERE
  Slice8 : xbsIndex_r4/Slice  nbits=1  boolean_output=on  mode=Lower Bit Location + Width  bit1=0  base1=MSB of Input  bit0=8  base0=LSB of Input  dbl_ovrd=off  block_type=slice
      settings: infoedit="Extracts a given range of bits from each input sample and presents it at the output.  The output type is ordinarily unsigned with binary point at zero, but can be Boolean when the slice is one bit wide.<P><P>Hardware notes: In hardware this block costs nothing."  block_version=VER_STRING_GOES_HERE
  Slice9 : xbsIndex_r4/Slice  nbits=1  boolean_output=on  mode=Lower Bit Location + Width  bit1=0  base1=MSB of Input  bit0=9  base0=LSB of Input  dbl_ovrd=off  block_type=slice
      settings: infoedit="Extracts a given range of bits from each input sample and presents it at the output.  The output type is ordinarily unsigned with binary point at zero, but can be Boolean when the slice is one bit wide.<P><P>Hardware notes: In hardware this block costs nothing."  block_version=VER_STRING_GOES_HERE
  tag scope: regRx_BER_reset=global, regRx_DivisionBypass=global, regRx_GlobalReset=global, regRx_dynPktEn=global, regRx_enExtPktDet=global, regRx_enIntPktDet=global, regRx_enableQuadCorrThresh=global, regRx_freqCorrBypass=global, regRx_freqCorr_TrainEn=global, regRx_freqCorr_lsEn=global, regRx_freqCorr_ssEn=global, regRx_newCoarseCFO=global, regRx_noSwitch_forceAntB=global, regRx_reqLongCorr=global, regRx_reqShortCorr=global, regRx_reqTwoLongCorr=global, regRx_resetOnBadHeader=global, regRx_simpleDynRxModulationEn=global, regRx_switchingDiv_En=global, regRx_useLongCorrCFOReset=global, regRx_usePilotArctan=global
  wires:
  Slice/1 -> [regRx_BER_reset]
  Slice1/1 -> Down Sample/1
  Slice2/1 -> [regRx_dynPktEn]
  Down Sample/1 -> [regRx_reqLongCorr]
  Slice4/1 -> Down Sample6/1
  Slice6/1 -> Down Sample1/1
  Slice5/1 -> Down Sample2/1
  Down Sample1/1 -> [regRx_reqTwoLongCorr]
  Down Sample2/1 -> [regRx_reqShortCorr]
  Slice7/1 -> Down Sample3/1
  Slice8/1 -> Down Sample4/1
  Down Sample3/1 -> [regRx_enExtPktDet]
  Down Sample4/1 -> [regRx_enIntPktDet]
  Slice9/1 -> Down Sample5/1
  Down Sample5/1 -> [regRx_freqCorrBypass]
  32b/1 -> Slice26/1, Slice14/1, Slice25/1, Slice15/1, Slice24/1, Slice22/1, Slice23/1, Slice13/1, Slice12/1, Slice11/1, Slice10/1, Slice9/1, Slice8/1, Slice7/1, Slice5/1, Slice6/1, Slice4/1, Slice3/1, Slice2/1, Slice1/1, Slice/1
  Slice10/1 -> Down Sample10/1
  Down Sample10/1 -> [regRx_freqCorr_lsEn]
  Slice11/1 -> Down Sample13/1
  Down Sample13/1 -> [regRx_freqCorr_ssEn]
  Down Sample14/1 -> [regRx_useLongCorrCFOReset]
  Slice12/1 -> Down Sample14/1
  Slice3/1 -> [regRx_simpleDynRxModulationEn]
  Slice13/1 -> [regRx_usePilotArctan]
  Slice22/1 -> Down Sample21/1
  Down Sample21/1 -> [regRx_freqCorr_TrainEn]
  Down Sample22/1 -> [regRx_DivisionBypass]
  Slice23/1 -> Down Sample22/1
  Slice24/1 -> Down Sample24/1
  Down Sample24/1 -> [regRx_switchingDiv_En]
  Slice25/1 -> [regRx_GlobalReset]
  Slice26/1 -> Down Sample26/1
  Down Sample26/1 -> [regRx_enableQuadCorrThresh]
  Down Sample6/1 -> [regRx_noSwitch_forceAntB]
  Down Sample7/1 -> [regRx_newCoarseCFO]
  Slice14/1 -> Down Sample7/1
  Down Sample8/1 -> [regRx_resetOnBadHeader]
  Slice15/1 -> Down Sample8/1
